simulink model "ofdm_txrx_mimo_2x"  (Simulink 6.6)
settings: Solver=VariableStepDiscrete  StartTime=0.0  StopTime=20e3  RelTol=1e-3
PreLoadFcn:
  | ofdm_rx_mimo_init
InitFcn:
  | ofdm_rx_mimo_init
StartFcn:
  | ofdm_rx_mimo_init
values from neighbouring files (symbols this model uses):
  BER_RAM_init_size = 2048    [ofdm_tx_mimo_init.m]
  CPLength = 16    [ofdm_tx_mimo_init.m]
  CRCPolynomial = hex2dec('04c11db7')    [ofdm_tx_mimo_init.m]
  freqOffset_track_filtCoef_i = hex2dec('2500')/2^32    [ofdm_rx_mimo_init.m]
  freqOffset_track_filtCoef_p = hex2dec('d0000')/2^32    [ofdm_rx_mimo_init.m]
  hi = zeros(1,length(cci))    [ofdm_rx_mimo_init.m]
  hr = zeros(1,length(ccr))    [ofdm_rx_mimo_init.m]
  max_CP_length = 16    [ofdm_tx_mimo_init.m]
  max_num_baseRateSymbols = 31    [ofdm_tx_mimo_init.m]
  max_num_subcarriers = 64    [ofdm_tx_mimo_init.m]
  max_num_trainingSymbols = 15    [ofdm_tx_mimo_init.m]
  max_numBytes = 16384    [ofdm_tx_mimo_init.m]
  max_OFDM_symbols = 2047    [ofdm_tx_mimo_init.m]
  modConstellation_qam16 = .75*[1 3 -1 -3]./3    [ofdm_tx_mimo_init.m]
  modConstellation_qam256 = 0.9375*[3 1 5 7 11 9 13 15 -3 -1 -5 -7 -11 -9 -13 -15]./15    [ofdm_tx_mimo_init.m]
  modConstellation_qam64 = 0.875*[3 1 5 7 -3 -1 -5 -7]./7    [ofdm_tx_mimo_init.m]
  modConstellation_qpsk = [1 -1]./sqrt(2)    [ofdm_tx_mimo_init.m]
  numBaseRateSymbols = 2    [ofdm_tx_mimo_init.m]
  numSubcarriers = 64    [ofdm_tx_mimo_init.m]
  numTrainingSymbols = 2    [ofdm_tx_mimo_init.m]
  pilot1_indicies = 7 + ( (64-7) * 2^16)    (= 3735559)    [ofdm_tx_mimo_init.m]
  pilot2_indicies = 21 + ( (64-21) * 2^16)    (= 2818069)    [ofdm_tx_mimo_init.m]
  pilotValue_neg = hex2dec('8000') + (2^16 * 0)    [ofdm_tx_mimo_init.m]
  pilotValue_pos = hex2dec('7FFF') + (2^16 * 0)    [ofdm_tx_mimo_init.m]
  pkt_crossCorr_thresh = 0.7    [ofdm_rx_mimo_init.m]
  pkt_energy_thresh = 0    [ofdm_rx_mimo_init.m]
  pkt_numPayloadBytes = 1400+42-6    (= 1436)    [ofdm_tx_mimo_init.m]
  pktByteNums = numHeaderBytes +  				byteIndex_numPayloadBytes(1)*2^8 +  				byteIndex_numPayloadBytes(2)*2^16 +  				byteIndex_simpleDynModMasks * 2^24    [ofdm_rx_mimo_init.m]
  PktDet_Delay = 26 + Symbol_Timing_Offset*2^7    (= 1050)    [ofdm_rx_mimo_init.m]
  preamble = 6*[0 shortsyms_10 longsyms_2]    [ofdm_tx_mimo_init.m]
  preamble_I = real(preamble)    [ofdm_tx_mimo_init.m]
  preamble_Q = imag(preamble)    [ofdm_tx_mimo_init.m]
  RAM_init_size = max_numBytes    (= 16384)    [ofdm_tx_mimo_init.m]
  reg_Interrupt_PktBuf_Control = reg_InterruptControl +  	1 * 2^16 +  	0 * 2^24    (= 65656)    [ofdm_rx_mimo_init.m]
  rx_controlBits = 1 * 2  		 + 1 * 4  		 + 0 * 8  		 + 1 * 16 * rx_SISO_Mode  		 + 1 * 32  		 + 1 * 64  		 + 0 * 128  		 + 1 * 256  		 + 0 * 512  		 + 1 * 1024  		 + 0 * 2048  		 + 1 * 4096  		 + 1 * 8192  		 + 0 * 16384  		 + 1 * 65536  		 + 1 * 131072  		 + 0 * 262144  		 + 1 * 524288    [ofdm_rx_mimo_init.m]
  Rx_PhaseNoiseTrack_K = 0.6094    [ofdm_rx_mimo_init.m]
  rx_postEq_scaling = round(rxScaling*2^11) * (1 + 2^16)    [ofdm_rx_mimo_init.m]
  RxDataScrambling_Seq = [40 198 78 63 82 173 102 245 48 111 172 115 147 230 216 93 72 65 62 2 205 242 122 90 128 83 105 97 73 10 5 252]    [ofdm_tx_mimo_init.m]
  simOnly_numSamples = length(preamble)+( (numSubcarriers+CPLength)*(numTrainingSymbols + numBaseRateSymbols + numFullRateSymbols/2) )    [ofdm_tx_mimo_init.m]
  train = [train train]    [ofdm_tx_mimo_init.m]
  tx_controlBits = (antB_preambleShift * 2^4) + (2^0 * tx_SISO_Mode) + 4*0 + 8*1    (= 73)    [ofdm_tx_mimo_init.m]
  TxDataScrambling_Seq = [40 198 78 63 82 173 102 245 48 111 172 115 147 230 216 93 72 65 62 2 205 242 122 90 128 83 105 97 73 10 5 252]    [ofdm_tx_mimo_init.m]
  txReg_symbolCounts = (2^16 * numFullRateSymbols) + (2^8 *numBaseRateSymbols ) + numTrainingSymbols    [ofdm_tx_mimo_init.m]
  TxRx_FFTScaling = bin2dec('010111') + (bin2dec('000101') * 2^6)    [ofdm_tx_mimo_init.m]
undefined symbols (not defined in the file or in its folder): AntA, AntB, BaseRate, CRC_table_gen, gcbh, intfilt, pktTiming_count_payloadStart, pktTiming_symbol_length, xlBlockLoadCallback, xlGetSimulinkPeriod
libraries: xbsIndex_r4 x3973, dspsigops x4, commchan3 x2, commrflib2 x2, dspsrcs4 x1
(part 22 of 38)

== system ofdm_txrx_mimo_2x/OFDM Rx MIMO/Equalizer & Packetizer/Equalizer/YB*[-HBA,HAA]/ComplexMult_x1 ==
  Re[A] : Inport
  Im[A] : Inport  Port=2
  Re[B] : Inport  Port=3
  Im[B] : Inport  Port=4
  Add1 : xbsIndex_r4/AddSub  mode=Addition  use_carryin=off  use_carryout=off  en=off  latency=0  precision=User Defined  arith_type="Signed  (2's comp)"  n_bits=18  bin_pt=16  quantization=Truncate  overflow=Flag as error  dbl_ovrd=off  use_behavioral_HDL=on  pipelined=off  use_rpm=on  hw_selection=Fabric  block_type=addsub
      settings: xl_area=[9 0 0 16 0 0 0]  block_version=VER_STRING_GOES_HERE
  Add2 : xbsIndex_r4/AddSub  mode=Addition  use_carryin=off  use_carryout=off  en=off  latency=0  precision=User Defined  arith_type="Signed  (2's comp)"  n_bits=18  bin_pt=16  quantization=Truncate  overflow=Flag as error  dbl_ovrd=off  use_behavioral_HDL=on  pipelined=off  use_rpm=on  hw_selection=Fabric  block_type=addsub
      settings: xl_area=[9 0 0 16 0 0 0]  block_version=VER_STRING_GOES_HERE
  Counter1 : xbsIndex_r4/Counter  Orientation=down  cnt_type=Free Running  cnt_to=4  operation=Up  start_count=0  cnt_by_val=1  arith_type=Unsigned  n_bits=1  bin_pt=0  load_pin=off  rst=off  en=off  explicit_period=on  period=1  dbl_ovrd=off  use_behavioral_HDL=off  use_rpm=on  implementation=Fabric  block_type=counter
      settings: xl_area=[3 3 0 4 0 0 0]  block_version=VER_STRING_GOES_HERE
  Delay3 : xbsIndex_r4/Delay  en=off  latency=1  dbl_ovrd=off  reg_retiming=off  block_type=delay
      settings: infoedit=Hardware notes: A delay line is a chain, each link of which is an SRL16 followed by a flip-flop. If register retiming is enabled, the delay line is a chain of flip-flops.  xl_area=[8 16 0 0 0 0 0]  block_version=VER_STRING_GOES_HERE
  Delay5 : xbsIndex_r4/Delay  en=off  latency=1  dbl_ovrd=off  reg_retiming=off  block_type=delay
      settings: infoedit=Hardware notes: A delay line is a chain, each link of which is an SRL16 followed by a flip-flop. If register retiming is enabled, the delay line is a chain of flip-flops.  xl_area=[8 16 0 0 0 0 0]  block_version=VER_STRING_GOES_HERE
  Down Sample1 : xbsIndex_r4/Down Sample  sample_ratio=2  sample_phase=First Value of Frame  en=off  latency=1  dbl_ovrd=off  block_type=dsamp
      settings: xl_area=[9 17 0 0 0 0 0]  block_version=VER_STRING_GOES_HERE
  Down Sample3 : xbsIndex_r4/Down Sample  sample_ratio=2  sample_phase=First Value of Frame  en=off  latency=1  dbl_ovrd=off  block_type=dsamp
      settings: xl_area=[9 17 0 0 0 0 0]  block_version=VER_STRING_GOES_HERE
  Mult3 : xbsIndex_r4/Mult  precision=User Defined  arith_type="Signed  (2's comp)"  n_bits=19  bin_pt=17  quantization=Truncate  overflow=Flag as error  en=off  latency=1  dbl_ovrd=off  use_behavioral_HDL=off  use_embedded=on  opt=Speed  optimum_pipeline=off  pipeline=off  use_rpm=on  placement_style=Rectangular shape  block_type=mult
      settings: infoedit=Hardware notes: To use the internal pipeline stage of the dedicated multiplier you must select 'Pipeline for maximum performance'.  xl_area=[14 27 0 27 0 1 0]  block_version=VER_STRING_GOES_HERE
  Mult4 : xbsIndex_r4/Mult  precision=User Defined  arith_type="Signed  (2's comp)"  n_bits=19  bin_pt=17  quantization=Truncate  overflow=Flag as error  en=off  latency=1  dbl_ovrd=off  use_behavioral_HDL=off  use_embedded=on  opt=Speed  optimum_pipeline=off  pipeline=off  use_rpm=on  placement_style=Rectangular shape  block_type=mult
      settings: infoedit=Hardware notes: To use the internal pipeline stage of the dedicated multiplier you must select 'Pipeline for maximum performance'.  xl_area=[14 27 0 27 0 1 0]  block_version=VER_STRING_GOES_HERE
  Mux{2..5} (4 alike) : xbsIndex_r4/Mux  inputs=2  en=off  latency=0  precision=Full  arith_type="Signed  (2's comp)"  n_bits=16  bin_pt=13  quantization=Truncate  overflow=Wrap  dbl_ovrd=off  block_type=mux
      settings: xl_area=[14 0 0 28 0 0 0]  block_version=VER_STRING_GOES_HERE
  Negate2 : xbsIndex_r4/Negate  precision=Full  arith_type="Signed  (2's comp)"  n_bits=14  bin_pt=13  quantization=Truncate  overflow=Flag as error  en=off  latency=0  dbl_ovrd=off  block_type=negate
      settings: xl_area=[7 0 0 14 0 0 0]  block_version=VER_STRING_GOES_HERE
  Up Sample{5..8} (4 alike) : xbsIndex_r4/Up Sample  sample_ratio=2  copy_samples=on  dbl_ovrd=off  block_type=usamp
      settings: infoedit="Up samples input data.  Inserted values  can be zeros or copies of the most recent input sample.<P><P>Hardware notes: No hardware is needed if inserted values are copies of the input sample; otherwise, a mux and single bit flip-flop are used."  xl_area=[0 0 0 0 0 0 0]  block_version=VER_STRING_GOES_HERE
  Re : Outport
  Im : Outport  Port=2
  wires:
  Counter1/1 -> Mux3/1, Mux2/1, Mux5/1, Mux4/1
  Delay3/1 -> Add2/2
  Mult3/1 -> Add2/1, Delay3/1
  Up Sample5/1 -> Negate2/1, Mux5/3
  Up Sample6/1 -> Mux2/3, Mux4/2
  Mux3/1 -> Mult3/1
  Mux2/1 -> Mult3/2
  Mux5/1 -> Mult4/1
  Mux4/1 -> Mult4/2
  Up Sample7/1 -> Mux3/2, Mux5/2
  Up Sample8/1 -> Mux2/2, Mux4/3
  Re[A]/1 -> Up Sample7/1
  Im[A]/1 -> Up Sample5/1
  Re[B]/1 -> Up Sample8/1
  Im[B]/1 -> Up Sample6/1
  Negate2/1 -> Mux3/3
  Mult4/1 -> Delay5/1, Add1/1
  Delay5/1 -> Add1/2
  Add1/1 -> Down Sample1/1
  Add2/1 -> Down Sample3/1
  Down Sample3/1 -> Re/1
  Down Sample1/1 -> Im/1

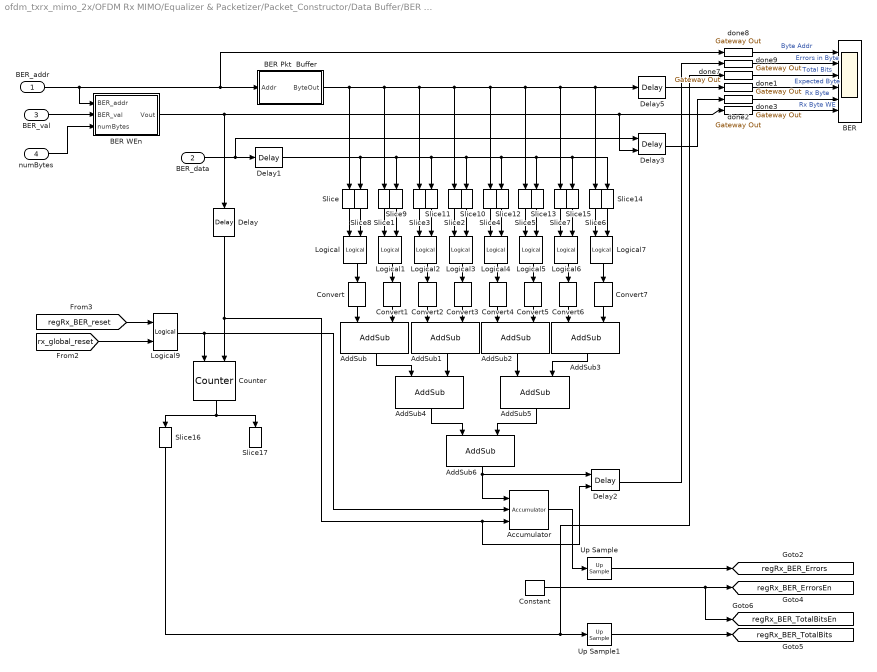
== system ofdm_txrx_mimo_2x/OFDM Rx MIMO/Equalizer & Packetizer/Packet_Constructor/Data Buffer/BER Calc ==
  BER_addr : Inport
  BER_data : Inport  Port=2
  BER_val : Inport  Port=3
  numBytes : Inport  Port=4
  Accumulator : xbsIndex_r4/Accumulator  operation=Add  n_bits=32  overflow=Wrap  scale=1  rst=on  hasbypass=off  en=on  dbl_ovrd=off  use_behavioral_HDL=on  implementation=Fabric  block_type=accum
      settings: infoedit="Adder or subtractor-based accumulator.   Output type and binary point position match the input.<P><P>Hardware notes: When \"Reinitialize with input 'b' on reset\" is selected, the accumulator is forced to run at the system rate even if the input 'b' is running at a slower rate."  xl_area=[0, 0, 0, 0, 0, 0, 0]  block_version=VER_STRING_GOES_HERE
  AddSub{,1..6} (7 alike) : xbsIndex_r4/AddSub  Orientation=down  mode=Addition  use_carryin=off  use_carryout=off  en=off  latency=0  precision=Full  arith_type=Unsigned  n_bits=8  bin_pt=2  quantization=Truncate  overflow=Wrap  dbl_ovrd=off  use_behavioral_HDL=on  pipelined=off  use_rpm=on  hw_selection=Fabric  block_type=addsub
      settings: xl_area=[0, 0, 0, 0, 0, 0, 0]  block_version=VER_STRING_GOES_HERE
  BER : Scope  NumInputPorts=6
      settings: TimeRange=7000  YMin=0~0~0~0~0~0  YMax=35~4~250~70~250~1  SaveName=ScopeData57
  BER Pkt  Buffer : Subsystem
  BER WEn : Subsystem
  Constant : xbsIndex_r4/Constant  arith_type=Boolean  const=1  n_bits=16  bin_pt=14  explicit_period=off  period=1  equ=P=C  opselect=C  inp2=PCIN>>17  opr=+  inp1=P  carry=CIN  dbl_ovrd=off  block_type=constant
      settings: block_version=VER_STRING_GOES_HERE
  Convert{,1..7} (8 alike) : xbsIndex_r4/Convert  Orientation=down  arith_type=Unsigned  n_bits=1  bin_pt=0  quantization=Truncate  overflow=Wrap  latency=0  dbl_ovrd=off  pipeline=off  block_type=convert
      settings: xl_area=[0, 0, 0, 0, 0, 0, 0]  block_version=VER_STRING_GOES_HERE
  Counter : xbsIndex_r4/Counter  Orientation=down  cnt_type=Free Running  cnt_to=Inf  operation=Up  start_count=0  cnt_by_val=8  arith_type=Unsigned  n_bits=48  bin_pt=0  load_pin=off  rst=on  en=on  explicit_period=off  period=1  dbl_ovrd=off  use_behavioral_HDL=off  use_rpm=on  implementation=Fabric  block_type=counter
      settings: xl_area=[0, 0, 0, 0, 0, 0, 0]  block_version=VER_STRING_GOES_HERE
  Delay : xbsIndex_r4/Delay  Orientation=down  en=off  latency=1  dbl_ovrd=off  reg_retiming=off  block_type=delay
      settings: infoedit=Hardware notes: A delay line is a chain, each link of which is an SRL16 followed by a flip-flop. If register retiming is enabled, the delay line is a chain of flip-flops.  xl_area=[0, 0, 0, 0, 0, 0, 0]  block_version=VER_STRING_GOES_HERE
  Delay1 : xbsIndex_r4/Delay  en=off  latency=1  dbl_ovrd=off  reg_retiming=off  block_type=delay
      settings: infoedit=Hardware notes: A delay line is a chain, each link of which is an SRL16 followed by a flip-flop. If register retiming is enabled, the delay line is a chain of flip-flops.  xl_area=[0, 0, 0, 0, 0, 0, 0]  block_version=VER_STRING_GOES_HERE
  Delay2 : xbsIndex_r4/Delay  en=on  latency=1  dbl_ovrd=off  reg_retiming=off  block_type=delay
      settings: infoedit=Hardware notes: A delay line is a chain, each link of which is an SRL16 followed by a flip-flop. If register retiming is enabled, the delay line is a chain of flip-flops.  xl_area=[0, 0, 0, 0, 0, 0, 0]  block_version=VER_STRING_GOES_HERE
  Delay3 : xbsIndex_r4/Delay  en=on  latency=1  dbl_ovrd=off  reg_retiming=off  block_type=delay
      settings: infoedit=Hardware notes: A delay line is a chain, each link of which is an SRL16 followed by a flip-flop. If register retiming is enabled, the delay line is a chain of flip-flops.  xl_area=[0, 0, 0, 0, 0, 0, 0]  block_version=VER_STRING_GOES_HERE
  Delay5 : xbsIndex_r4/Delay  en=off  latency=1  dbl_ovrd=off  reg_retiming=off  block_type=delay
      settings: infoedit=Hardware notes: A delay line is a chain, each link of which is an SRL16 followed by a flip-flop. If register retiming is enabled, the delay line is a chain of flip-flops.  xl_area=[0, 0, 0, 0, 0, 0, 0]  block_version=VER_STRING_GOES_HERE
  Logical{,1..7} (8 alike) : xbsIndex_r4/Logical  Orientation=down  logical_function=XOR  inputs=2  en=off  latency=0  precision=Full  arith_type=Unsigned  n_bits=1  bin_pt=0  align_bp=on  dbl_ovrd=off  block_type=logical
      settings: xl_area=[0, 0, 0, 0, 0, 0, 0]  block_version=VER_STRING_GOES_HERE
  Logical9 : xbsIndex_r4/Logical  logical_function=OR  inputs=2  en=off  latency=0  precision=Full  arith_type=Unsigned  n_bits=8  bin_pt=2  align_bp=on  dbl_ovrd=off  block_type=logical
      settings: xl_area=[4 0 0 4 0 0 0]  block_version=VER_STRING_GOES_HERE
  Slice : xbsIndex_r4/Slice  Orientation=down  nbits=1  boolean_output=on  mode=Upper Bit Location + Width  bit1=0  base1=MSB of Input  bit0=0  base0=LSB of Input  dbl_ovrd=off  block_type=slice
      settings: infoedit="Extracts a given range of bits from each input sample and presents it at the output.  The output type is ordinarily unsigned with binary point at zero, but can be Boolean when the slice is one bit wide.<P><P>Hardware notes: In hardware this block costs nothing."  block_version=VER_STRING_GOES_HERE
  Slice1 : xbsIndex_r4/Slice  Orientation=down  nbits=1  boolean_output=on  mode=Upper Bit Location + Width  bit1=-1  base1=MSB of Input  bit0=0  base0=LSB of Input  dbl_ovrd=off  block_type=slice
      settings: infoedit="Extracts a given range of bits from each input sample and presents it at the output.  The output type is ordinarily unsigned with binary point at zero, but can be Boolean when the slice is one bit wide.<P><P>Hardware notes: In hardware this block costs nothing."  block_version=VER_STRING_GOES_HERE
  Slice10 : xbsIndex_r4/Slice  Orientation=down  nbits=1  boolean_output=on  mode=Upper Bit Location + Width  bit1=-3  base1=MSB of Input  bit0=0  base0=LSB of Input  dbl_ovrd=off  block_type=slice
      settings: infoedit="Extracts a given range of bits from each input sample and presents it at the output.  The output type is ordinarily unsigned with binary point at zero, but can be Boolean when the slice is one bit wide.<P><P>Hardware notes: In hardware this block costs nothing."  block_version=VER_STRING_GOES_HERE
  Slice11 : xbsIndex_r4/Slice  Orientation=down  nbits=1  boolean_output=on  mode=Upper Bit Location + Width  bit1=-2  base1=MSB of Input  bit0=0  base0=LSB of Input  dbl_ovrd=off  block_type=slice
      settings: infoedit="Extracts a given range of bits from each input sample and presents it at the output.  The output type is ordinarily unsigned with binary point at zero, but can be Boolean when the slice is one bit wide.<P><P>Hardware notes: In hardware this block costs nothing."  block_version=VER_STRING_GOES_HERE
  Slice12 : xbsIndex_r4/Slice  Orientation=down  nbits=1  boolean_output=on  mode=Upper Bit Location + Width  bit1=-4  base1=MSB of Input  bit0=0  base0=LSB of Input  dbl_ovrd=off  block_type=slice
      settings: infoedit="Extracts a given range of bits from each input sample and presents it at the output.  The output type is ordinarily unsigned with binary point at zero, but can be Boolean when the slice is one bit wide.<P><P>Hardware notes: In hardware this block costs nothing."  block_version=VER_STRING_GOES_HERE
  Slice13 : xbsIndex_r4/Slice  Orientation=down  nbits=1  boolean_output=on  mode=Upper Bit Location + Width  bit1=-5  base1=MSB of Input  bit0=0  base0=LSB of Input  dbl_ovrd=off  block_type=slice
      settings: infoedit="Extracts a given range of bits from each input sample and presents it at the output.  The output type is ordinarily unsigned with binary point at zero, but can be Boolean when the slice is one bit wide.<P><P>Hardware notes: In hardware this block costs nothing."  block_version=VER_STRING_GOES_HERE
  Slice14 : xbsIndex_r4/Slice  Orientation=down  nbits=1  boolean_output=on  mode=Upper Bit Location + Width  bit1=-7  base1=MSB of Input  bit0=0  base0=LSB of Input  dbl_ovrd=off  block_type=slice
      settings: infoedit="Extracts a given range of bits from each input sample and presents it at the output.  The output type is ordinarily unsigned with binary point at zero, but can be Boolean when the slice is one bit wide.<P><P>Hardware notes: In hardware this block costs nothing."  block_version=VER_STRING_GOES_HERE
  Slice15 : xbsIndex_r4/Slice  Orientation=down  nbits=1  boolean_output=on  mode=Upper Bit Location + Width  bit1=-6  base1=MSB of Input  bit0=0  base0=LSB of Input  dbl_ovrd=off  block_type=slice
      settings: infoedit="Extracts a given range of bits from each input sample and presents it at the output.  The output type is ordinarily unsigned with binary point at zero, but can be Boolean when the slice is one bit wide.<P><P>Hardware notes: In hardware this block costs nothing."  block_version=VER_STRING_GOES_HERE
  Slice16 : xbsIndex_r4/Slice  Orientation=down  nbits=32  boolean_output=off  mode=Lower Bit Location + Width  bit1=0  base1=MSB of Input  bit0=0  base0=LSB of Input  dbl_ovrd=off  block_type=slice
      settings: infoedit="Extracts a given range of bits from each input sample and presents it at the output.  The output type is ordinarily unsigned with binary point at zero, but can be Boolean when the slice is one bit wide.<P><P>Hardware notes: In hardware this block costs nothing."  block_version=VER_STRING_GOES_HERE
  Slice17 : xbsIndex_r4/Slice  Orientation=down  nbits=16  boolean_output=off  mode=Upper Bit Location + Width  bit1=0  base1=MSB of Input  bit0=0  base0=LSB of Input  dbl_ovrd=off  block_type=slice
      settings: infoedit="Extracts a given range of bits from each input sample and presents it at the output.  The output type is ordinarily unsigned with binary point at zero, but can be Boolean when the slice is one bit wide.<P><P>Hardware notes: In hardware this block costs nothing."  block_version=VER_STRING_GOES_HERE
  Slice2 : xbsIndex_r4/Slice  Orientation=down  nbits=1  boolean_output=on  mode=Upper Bit Location + Width  bit1=-3  base1=MSB of Input  bit0=0  base0=LSB of Input  dbl_ovrd=off  block_type=slice
      settings: infoedit="Extracts a given range of bits from each input sample and presents it at the output.  The output type is ordinarily unsigned with binary point at zero, but can be Boolean when the slice is one bit wide.<P><P>Hardware notes: In hardware this block costs nothing."  block_version=VER_STRING_GOES_HERE
  Slice3 : xbsIndex_r4/Slice  Orientation=down  nbits=1  boolean_output=on  mode=Upper Bit Location + Width  bit1=-2  base1=MSB of Input  bit0=0  base0=LSB of Input  dbl_ovrd=off  block_type=slice
      settings: infoedit="Extracts a given range of bits from each input sample and presents it at the output.  The output type is ordinarily unsigned with binary point at zero, but can be Boolean when the slice is one bit wide.<P><P>Hardware notes: In hardware this block costs nothing."  block_version=VER_STRING_GOES_HERE
  Slice4 : xbsIndex_r4/Slice  Orientation=down  nbits=1  boolean_output=on  mode=Upper Bit Location + Width  bit1=-4  base1=MSB of Input  bit0=0  base0=LSB of Input  dbl_ovrd=off  block_type=slice
      settings: infoedit="Extracts a given range of bits from each input sample and presents it at the output.  The output type is ordinarily unsigned with binary point at zero, but can be Boolean when the slice is one bit wide.<P><P>Hardware notes: In hardware this block costs nothing."  block_version=VER_STRING_GOES_HERE
  Slice5 : xbsIndex_r4/Slice  Orientation=down  nbits=1  boolean_output=on  mode=Upper Bit Location + Width  bit1=-5  base1=MSB of Input  bit0=0  base0=LSB of Input  dbl_ovrd=off  block_type=slice
      settings: infoedit="Extracts a given range of bits from each input sample and presents it at the output.  The output type is ordinarily unsigned with binary point at zero, but can be Boolean when the slice is one bit wide.<P><P>Hardware notes: In hardware this block costs nothing."  block_version=VER_STRING_GOES_HERE
  Slice6 : xbsIndex_r4/Slice  Orientation=down  nbits=1  boolean_output=on  mode=Upper Bit Location + Width  bit1=-7  base1=MSB of Input  bit0=0  base0=LSB of Input  dbl_ovrd=off  block_type=slice
      settings: infoedit="Extracts a given range of bits from each input sample and presents it at the output.  The output type is ordinarily unsigned with binary point at zero, but can be Boolean when the slice is one bit wide.<P><P>Hardware notes: In hardware this block costs nothing."  block_version=VER_STRING_GOES_HERE
  Slice7 : xbsIndex_r4/Slice  Orientation=down  nbits=1  boolean_output=on  mode=Upper Bit Location + Width  bit1=-6  base1=MSB of Input  bit0=0  base0=LSB of Input  dbl_ovrd=off  block_type=slice
      settings: infoedit="Extracts a given range of bits from each input sample and presents it at the output.  The output type is ordinarily unsigned with binary point at zero, but can be Boolean when the slice is one bit wide.<P><P>Hardware notes: In hardware this block costs nothing."  block_version=VER_STRING_GOES_HERE
  Slice8 : xbsIndex_r4/Slice  Orientation=down  nbits=1  boolean_output=on  mode=Upper Bit Location + Width  bit1=0  base1=MSB of Input  bit0=0  base0=LSB of Input  dbl_ovrd=off  block_type=slice
      settings: infoedit="Extracts a given range of bits from each input sample and presents it at the output.  The output type is ordinarily unsigned with binary point at zero, but can be Boolean when the slice is one bit wide.<P><P>Hardware notes: In hardware this block costs nothing."  block_version=VER_STRING_GOES_HERE
  Slice9 : xbsIndex_r4/Slice  Orientation=down  nbits=1  boolean_output=on  mode=Upper Bit Location + Width  bit1=-1  base1=MSB of Input  bit0=0  base0=LSB of Input  dbl_ovrd=off  block_type=slice
      settings: infoedit="Extracts a given range of bits from each input sample and presents it at the output.  The output type is ordinarily unsigned with binary point at zero, but can be Boolean when the slice is one bit wide.<P><P>Hardware notes: In hardware this block costs nothing."  block_version=VER_STRING_GOES_HERE
  Up Sample : xbsIndex_r4/Up Sample  sample_ratio=2  copy_samples=on  dbl_ovrd=off  block_type=usamp
      settings: infoedit="Up samples input data.  Inserted values  can be zeros or copies of the most recent input sample.<P><P>Hardware notes: No hardware is needed if inserted values are copies of the input sample; otherwise, a mux and single bit flip-flop are used."  block_version=VER_STRING_GOES_HERE
  Up Sample1 : xbsIndex_r4/Up Sample  sample_ratio=2  copy_samples=on  dbl_ovrd=off  block_type=usamp
      settings: infoedit="Up samples input data.  Inserted values  can be zeros or copies of the most recent input sample.<P><P>Hardware notes: No hardware is needed if inserted values are copies of the input sample; otherwise, a mux and single bit flip-flop are used."  block_version=VER_STRING_GOES_HERE
  done{1..3,7..9} (6 alike) : xbsIndex_r4/Gateway Out  hdl_port=off  timing_constraint=None  locs_specified=off  LOCs={}  block_type=gatewayout
      settings: infoedit="Gateway out block.  Converts Xilinx fixed point inputs into ouputs of type Simulink integer, double, or fixed point.<P><P>Hardware notes:  In hardware these blocks become top level output ports or are discarded, depending on how they are configured."  xl_area=[0 0 0 0 1 0 0]  block_version=VER_STRING_GOES_HERE
  tag scope: regRx_BER_Errors=global, regRx_BER_ErrorsEn=global, regRx_BER_TotalBits=global, regRx_BER_TotalBitsEn=global
  wires:
  Delay/1 -> Counter/2, Accumulator/3, Delay2/2
  Slice16/1 -> Up Sample1/1, done7/1
  Counter/1 -> Slice17/1, Slice16/1
  BER WEn/1(Vout) -> done3/1, Delay3/2, Delay/1
  Convert7/1 -> AddSub3/2
  Convert6/1 -> AddSub3/1
  Convert5/1 -> AddSub2/2
  Convert4/1 -> AddSub2/1
  Convert3/1 -> AddSub1/2
  Convert2/1 -> AddSub1/1
  Convert1/1 -> AddSub/2
  Convert/1 -> AddSub/1
  Accumulator/1 -> Up Sample/1
  AddSub6/1 -> Accumulator/1, Delay2/1
  AddSub5/1 -> AddSub6/2
  AddSub4/1 -> AddSub6/1
  AddSub1/1 -> AddSub4/2
  AddSub/1 -> AddSub4/1
  AddSub3/1 -> AddSub5/2
  AddSub2/1 -> AddSub5/1
  Logical7/1 -> Convert7/1
  Logical6/1 -> Convert6/1
  Logical5/1 -> Convert5/1
  Logical4/1 -> Convert4/1
  Logical3/1 -> Convert3/1
  Logical2/1 -> Convert2/1
  Logical1/1 -> Convert1/1
  Logical/1 -> Convert/1
  Slice14/1 -> Logical7/2
  Slice6/1 -> Logical7/1
  Slice15/1 -> Logical6/2
  Slice7/1 -> Logical6/1
  Slice13/1 -> Logical5/2
  Slice5/1 -> Logical5/1
  Slice12/1 -> Logical4/2
  Slice4/1 -> Logical4/1
  Slice10/1 -> Logical3/2
  Slice2/1 -> Logical3/1
  Slice11/1 -> Logical2/2
  Slice3/1 -> Logical2/1
  Slice9/1 -> Logical1/2
  Slice1/1 -> Logical1/1
  Slice8/1 -> Logical/2
  Slice/1 -> Logical/1
  BER_data/1 -> Delay1/1, Delay3/1
  BER_addr/1 -> done8/1, BER Pkt  Buffer/1(Addr), BER WEn/1(BER_addr)
  [regRx_BER_reset] -> Logical9/1
  Logical9/1 -> Counter/1, Accumulator/2
  [rx_global_reset] -> Logical9/2
  Constant/1 -> [regRx_BER_ErrorsEn], [regRx_BER_TotalBitsEn]
  Up Sample/1 -> [regRx_BER_Errors]
  Up Sample1/1 -> [regRx_BER_TotalBits]
  Delay1/1 -> Slice11/1, Slice15/1, Slice14/1, Slice13/1, Slice12/1, Slice10/1, Slice9/1, Slice8/1
  Delay2/1 -> done9/1
  BER Pkt  Buffer/1(ByteOut) -> Slice1/1, Slice3/1, Slice2/1, Slice4/1, Slice5/1, Slice7/1, Slice6/1, Delay5/1, Slice/1
  done8/1 -> BER/1  "Byte Addr"
  done9/1 -> BER/2  "Errors in Byte"
  done7/1 -> BER/3  "Total Bits"
  done1/1 -> BER/4  "Expected Byte"
  done2/1 -> BER/5  "Rx Byte"
  done3/1 -> BER/6  "Rx Byte WE"
  Delay3/1 -> done2/1
  Delay5/1 -> done1/1
  BER_val/1 -> BER WEn/2(BER_val)
  numBytes/1 -> BER WEn/3(numBytes)

== system ofdm_txrx_mimo_2x/OFDM Rx MIMO/Equalizer & Packetizer/Packet_Constructor/Data Buffer/Descrambling & Pkt Buffer ==
  Addr : Inport
  Data : Inport  Port=2
  We : Inport  Port=3
  BRAM WEn Decoding : Subsystem
  DataReplication : Subsystem
  De-Scrambling : Subsystem
  Error Injector (Sim Only!) : Subsystem
  Gateway Out{1,3..5} (4 alike) : xbsIndex_r4/Gateway Out  hdl_port=off  timing_constraint=None  locs_specified=off  LOCs={}  block_type=gatewayout
      settings: infoedit="Gateway out block.  Converts Xilinx fixed point inputs into ouputs of type Simulink integer, double, or fixed point.<P><P>Hardware notes:  In hardware these blocks become top level output ports or are discarded, depending on how they are configured."  xl_area=[0, 0, 0, 0, 0, 0, 0]  block_version=VER_STRING_GOES_HERE
  MSB : xbsIndex_r4/Slice  nbits=log2(max_numBytes)-3  boolean_output=off  mode=Upper Bit Location + Width  bit1=0  base1=MSB of Input  bit0=0  base0=LSB of Input  dbl_ovrd=off  block_type=slice
      settings: infoedit="Extracts a given range of bits from each input sample and presents it at the output.  The output type is ordinarily unsigned with binary point at zero, but can be Boolean when the slice is one bit wide.<P><P>Hardware notes: In hardware this block costs nothing."  block_version=8.2.02
  Rx Data Descrambling : Scope  NumInputPorts=6
      settings: YMin=181~0~0~0~0~-1  YMax=192~275~1~275~250~1  SaveName=ScopeData27
  Up Sample{3..7} (5 alike) : xbsIndex_r4/Up Sample  sample_ratio=2  copy_samples=on  dbl_ovrd=off  block_type=usamp
      settings: infoedit="Up samples input data.  Inserted values  can be zeros or copies of the most recent input sample.<P><P>Hardware notes: No hardware is needed if inserted values are copies of the input sample; otherwise, a mux and single bit flip-flop are used."  block_version=8.2.02
  " Addr" : Outport
  " Data" : Outport  Port=2
  " Vout" : Outport  Port=3
  tag scope: BRAM_Rx_AnyWrEn=global, BRAM_Rx_DOut=global, BRAM_Rx_WrAddr=global, BRAM_Rx_WrEn=global, DebugBRAM_Rx_ByteOut=global
  wires:
  De-Scrambling/1(" Addr") -> Up Sample5/1, Up Sample7/1, " Addr"/1, Error Injector (Sim Only!)/1(ByteAddr)
  De-Scrambling/2(" Data") -> Gateway Out5/1, Up Sample3/1, " Data"/1, Error Injector (Sim Only!)/2(Data_I)
  De-Scrambling/3(" We") -> " Vout"/1, Up Sample4/1, Up Sample6/1
  We/1 -> Gateway Out4/1, De-Scrambling/3(We)
  Data/1 -> Gateway Out3/1, De-Scrambling/2(Data)
  Addr/1 -> Gateway Out1/1, De-Scrambling/1(Addr)
  Gateway Out1/1 -> Rx Data Descrambling/1
  Gateway Out3/1 -> Rx Data Descrambling/2
  Gateway Out4/1 -> Rx Data Descrambling/3
  Gateway Out5/1 -> Rx Data Descrambling/4
  DataReplication/1(8BytesOut) -> [BRAM_Rx_DOut]
  BRAM WEn Decoding/1(ByteEn) -> [BRAM_Rx_WrEn]
  MSB/1 -> [BRAM_Rx_WrAddr]
  Up Sample3/1 -> DataReplication/1(ByteIn), [DebugBRAM_Rx_ByteOut]
  Up Sample4/1 -> BRAM WEn Decoding/2(WrEn)
  Up Sample5/1 -> BRAM WEn Decoding/1(Byte Addr)
  Up Sample6/1 -> [BRAM_Rx_AnyWrEn]
  Up Sample7/1 -> MSB/1

== system ofdm_txrx_mimo_2x/OFDM Rx MIMO/Equalizer & Packetizer/Packet_Constructor/Data Buffer/Error Checking ==
  ByteIn : Inport
  Vin : Inport  Port=2
  ByteAddr : Inport  Port=3
  NumBytes : Inport  Port=4
  Rst : Inport  Port=5
  CRC Calc : Subsystem
  Error Checking : Subsystem
  Gateway Out{1..11} (11 alike) : xbsIndex_r4/Gateway Out  hdl_port=off  timing_constraint=None  locs_specified=off  LOCs={}  block_type=gatewayout
      settings: infoedit="Gateway out block.  Converts Xilinx fixed point inputs into ouputs of type Simulink integer, double, or fixed point.<P><P>Hardware notes:  In hardware these blocks become top level output ports or are discarded, depending on how they are configured."  xl_area=[0, 0, 0, 0, 0, 0, 0]  block_version=VER_STRING_GOES_HERE
  Posedge : Subsystem
  Relational : xbsIndex_r4/Relational  mode=a>=b  en=off  latency=0  dbl_ovrd=off  block_type=relational
      settings: xl_area=[0, 0, 0, 0, 0, 0, 0]  block_version=VER_STRING_GOES_HERE
  Rx CRC : Scope  NumInputPorts=6
      settings: YMin=0~0~-1~0~0~-1  YMax=175~1~1~4.5e+009~1~1  SaveName=ScopeData8
  Rx CRC Bytes : Scope  NumInputPorts=6
      settings: TimeRange=6000  YMin=0~0~0~0~-1~0  YMax=300~300~300~300~1~1  SaveName=ScopeData71
  PktError : Outport
  ResetRx : Outport  Port=2
  GoodHeader : Outport  Port=3
  BadHeader : Outport  Port=4
  wires:
  Vin/1 -> CRC Calc/2(Vin), Gateway Out11/1, Gateway Out1/1
  ByteIn/1 -> CRC Calc/1(ByteIn), Gateway Out6/1
  Rst/1 -> Error Checking/2(Rst), CRC Calc/3(Rst), Gateway Out3/1
  CRC Calc/1(32-bit CRC) -> Error Checking/1(32-bit CRC)
  Gateway Out1/1 -> Rx CRC/1
  Gateway Out3/1 -> Rx CRC/2
  Gateway Out4/1 -> Rx CRC/3
  Gateway Out5/1 -> Rx CRC/4
  Gateway Out2/1 -> Rx CRC/5
  Gateway Out11/1 -> Rx CRC Bytes/6
  ByteAddr/1 -> Relational/1, Gateway Out4/1, Error Checking/5(ByteAddr), Gateway Out8/1
  NumBytes/1 -> Relational/2, Gateway Out5/1, Error Checking/4(NumBytes), Gateway Out7/1
  Relational/1 -> Posedge/1(D)
  Posedge/1(Q) -> Error Checking/3(LastByte)
  Error Checking/1(PktError) -> PktError/1, Gateway Out2/1, Gateway Out9/1
  Gateway Out6/1 -> Rx CRC Bytes/1
  Gateway Out7/1 -> Rx CRC Bytes/2
  Gateway Out8/1 -> Rx CRC Bytes/3
  Gateway Out9/1 -> Rx CRC Bytes/4
  Gateway Out10/1 -> Rx CRC Bytes/5
  Error Checking/2(ResetRx) -> Gateway Out10/1, ResetRx/1
  Error Checking/3(GoodHeader) -> GoodHeader/1
  Error Checking/4(BadHeader) -> BadHeader/1

== system ofdm_txrx_mimo_2x/OFDM Rx MIMO/Equalizer & Packetizer/Packet_Constructor/Data Buffer/Header Decoding ==
  RxByteAddr : Inport
  RxByte : Inport  Port=2
  RxByteWE : Inport  Port=3
  Rst : Inport  Port=4
  4LSB : xbsIndex_r4/Slice  nbits=4  boolean_output=off  mode=Lower Bit Location + Width  bit1=0  base1=MSB of Input  bit0=0  base0=LSB of Input  dbl_ovrd=off  block_type=slice
      settings: infoedit="Extracts a given range of bits from each input sample and presents it at the output.  The output type is ordinarily unsigned with binary point at zero, but can be Boolean when the slice is one bit wide.<P><P>Hardware notes: In hardware this block costs nothing."  block_version=VER_STRING_GOES_HERE
  4MSB : xbsIndex_r4/Slice  nbits=4  boolean_output=off  mode=Upper Bit Location + Width  bit1=0  base1=MSB of Input  bit0=0  base0=LSB of Input  dbl_ovrd=off  block_type=slice
      settings: infoedit="Extracts a given range of bits from each input sample and presents it at the output.  The output type is ordinarily unsigned with binary point at zero, but can be Boolean when the slice is one bit wide.<P><P>Hardware notes: In hardware this block costs nothing."  block_version=VER_STRING_GOES_HERE
  8LSB : xbsIndex_r4/Slice  nbits=8  boolean_output=off  mode=Lower Bit Location + Width  bit1=0  base1=MSB of Input  bit0=0  base0=LSB of Input  dbl_ovrd=off  block_type=slice
      settings: infoedit="Extracts a given range of bits from each input sample and presents it at the output.  The output type is ordinarily unsigned with binary point at zero, but can be Boolean when the slice is one bit wide.<P><P>Hardware notes: In hardware this block costs nothing."  block_version=VER_STRING_GOES_HERE
  8LSB+8 : xbsIndex_r4/Slice  nbits=8  boolean_output=off  mode=Lower Bit Location + Width  bit1=0  base1=MSB of Input  bit0=8  base0=LSB of Input  dbl_ovrd=off  block_type=slice
      settings: infoedit="Extracts a given range of bits from each input sample and presents it at the output.  The output type is ordinarily unsigned with binary point at zero, but can be Boolean when the slice is one bit wide.<P><P>Hardware notes: In hardware this block costs nothing."  block_version=VER_STRING_GOES_HERE
  8MSB : xbsIndex_r4/Slice  nbits=8  boolean_output=off  mode=Upper Bit Location + Width  bit1=0  base1=MSB of Input  bit0=0  base0=LSB of Input  dbl_ovrd=off  block_type=slice
      settings: infoedit="Extracts a given range of bits from each input sample and presents it at the output.  The output type is ordinarily unsigned with binary point at zero, but can be Boolean when the slice is one bit wide.<P><P>Hardware notes: In hardware this block costs nothing."  block_version=VER_STRING_GOES_HERE
  Assert1 : xbsIndex_r4/Assert  assert_type=off  type_source=Explicitly  arith_type="Signed  (2's comp)"  n_bits=16  bin_pt=14  assert_rate=on  rate_source=Explicitly  period=2  output_port=on  block_type=assert
      settings: infoedit=Asserts a user-defined sample rate and/or type on a signal.<P><P>Hardware notes: In hardware this block costs nothing.  block_version=VER_STRING_GOES_HERE
  Assert2 : xbsIndex_r4/Assert  assert_type=off  type_source=Explicitly  arith_type="Signed  (2's comp)"  n_bits=16  bin_pt=14  assert_rate=on  rate_source=Explicitly  period=2  output_port=on  block_type=assert
      settings: infoedit=Asserts a user-defined sample rate and/or type on a signal.<P><P>Hardware notes: In hardware this block costs nothing.  block_version=VER_STRING_GOES_HERE
  Convert : xbsIndex_r4/Convert  arith_type=Boolean  n_bits=8  bin_pt=6  quantization=Truncate  overflow=Wrap  latency=0  dbl_ovrd=off  pipeline=off  block_type=convert
      settings: xl_area=[0, 0, 0, 0, 0, 0, 0]  block_version=VER_STRING_GOES_HERE
  Logical1 : xbsIndex_r4/Logical  logical_function=AND  inputs=2  en=off  latency=0  precision=Full  arith_type=Unsigned  n_bits=8  bin_pt=2  align_bp=on  dbl_ovrd=off  block_type=logical
      settings: xl_area=[1 0 0 1 0 0 0]  block_version=VER_STRING_GOES_HERE
  Logical4 : xbsIndex_r4/Logical  logical_function=AND  inputs=3  en=off  latency=0  precision=Full  arith_type=Unsigned  n_bits=8  bin_pt=2  align_bp=on  dbl_ovrd=off  block_type=logical
      settings: xl_area=[1 0 0 1 0 0 0]  block_version=VER_STRING_GOES_HERE
  Logical5 : xbsIndex_r4/Logical  logical_function=AND  inputs=3  en=off  latency=0  precision=Full  arith_type=Unsigned  n_bits=8  bin_pt=2  align_bp=on  dbl_ovrd=off  block_type=logical
      settings: xl_area=[1 0 0 1 0 0 0]  block_version=VER_STRING_GOES_HERE
  Register1 : xbsIndex_r4/Register  init=0%floor((pkt_numPayloadBytes/256))  rst=on  en=on  dbl_ovrd=off  block_type=register
      settings: xl_area=[5 10 0 0 0 0 0]  block_version=VER_STRING_GOES_HERE
  Relational1 : xbsIndex_r4/Relational  mode=a=b  en=off  latency=0  dbl_ovrd=off  block_type=relational
      settings: xl_area=[6 0 0 11 0 0 0]  block_version=VER_STRING_GOES_HERE
  Relational2 : xbsIndex_r4/Relational  mode=a=b  en=off  latency=0  dbl_ovrd=off  block_type=relational
      settings: xl_area=[6 0 0 11 0 0 0]  block_version=VER_STRING_GOES_HERE
  Relational3 : xbsIndex_r4/Relational  mode=a=b  en=off  latency=0  dbl_ovrd=off  block_type=relational
      settings: xl_area=[6 0 0 11 0 0 0]  block_version=VER_STRING_GOES_HERE
  Rx Bytes : Scope  NumInputPorts=5
      settings: TimeRange=1800  YMin=116~80~137.75~0~1.9  YMax=120~220~152.25~1~2.1  SaveName=ScopeData65
  done{1..10} (10 alike) : xbsIndex_r4/Gateway Out  hdl_port=off  timing_constraint=None  locs_specified=off  LOCs={}  block_type=gatewayout
      settings: infoedit="Gateway out block.  Converts Xilinx fixed point inputs into ouputs of type Simulink integer, double, or fixed point.<P><P>Hardware notes:  In hardware these blocks become top level output ports or are discarded, depending on how they are configured."  xl_area=[0 0 0 0 1 0 0]  block_version=VER_STRING_GOES_HERE
  modMasks : Display
  "numBytes " : Display
  numBytesUpdate : Subsystem
  numBytes_LSB : Display
  numBytes_MSB : Display
  numBytes_modMasks : Display
  numBytes : Outport
  modMask : Outport  Port=2
  wires:
  Convert/1 -> Logical5/3, Logical4/3
  [regRx_dynPktEn] -> Convert/1
  Relational3/1 -> Logical5/2
  8LSB+8/1 -> Relational3/2, done4/1
  Relational2/1 -> Logical4/2
  8LSB/1 -> Relational2/2, done3/1
  Rst/1 -> numBytesUpdate/1(rst), Register1/2
  Logical4/1 -> numBytesUpdate/3(lsb_EN)
  Logical5/1 -> numBytesUpdate/4(msb_EN)
  numBytesUpdate/1(numBytes) -> Assert1/1
  Assert1/1 -> numBytes/1, done1/1, done8/1
  [regRx_headerByteNums] -> 8LSB+8/1, 8LSB/1, 8MSB/1
  RxByteWE/1 -> Logical4/1, Logical5/1, Logical1/1, done2/1
  RxByte/1 -> numBytesUpdate/2(d), Register1/1, done7/1
  RxByteAddr/1 -> Relational2/1, Relational3/1, Relational1/1, done6/1
  Relational1/1 -> Logical1/2
  Logical1/1 -> Register1/3
  8MSB/1 -> Relational1/2, done5/1
  Register1/1 -> Assert2/1
  Assert2/1 -> modMask/1, 4MSB/1, 4LSB/1, done9/1, done10/1
  done6/1 -> Rx Bytes/1
  done7/1 -> Rx Bytes/2
  done1/1 -> Rx Bytes/3
  done9/1 -> Rx Bytes/5
  done2/1 -> Rx Bytes/4
  done3/1 -> numBytes_LSB/1
  done4/1 -> numBytes_MSB/1
  done5/1 -> numBytes_modMasks/1
  done8/1 -> "numBytes "/1
  done10/1 -> modMasks/1

== system ofdm_txrx_mimo_2x/OFDM Rx MIMO/Equalizer & Packetizer/Packet_Constructor/Modulation RAM/Addr Gen ==
  note: The modulation RAM stores 3x(numSubcarriers) values:
      | [0:numSc-1]: Masks for antenna A full rate symbols
      | [numSc:2*numSc-1]: Masks for antenna B full rate symbols
      | [2*numSc:3*numSc-1]: Masks for base rate symbols (same for both antennas)
  note: This block produces the correct memory address to lookup the modulation mask for a given subcarrier in a given OFDM symbol.
      | The LSB of the symbol index is treated as the antenna selector. During base rate symbols, this bit is forced to zero so that both
      | antennas use the same set of modulation masks for their base rate symbols.
  Xk_index : Inport
  Sym Index : Inport  Port=2
  1LSB : xbsIndex_r4/Slice  nbits=1  boolean_output=off  mode=Lower Bit Location + Width  bit1=0  base1=MSB of Input  bit0=0  base0=LSB of Input  dbl_ovrd=off  block_type=slice
      settings: infoedit="Extracts a given range of bits from each input sample and presents it at the output.  The output type is ordinarily unsigned with binary point at zero, but can be Boolean when the slice is one bit wide.<P><P>Hardware notes: In hardware this block costs nothing."  block_version=VER_STRING_GOES_HERE
  1b Zero : xbsIndex_r4/Constant  arith_type=Unsigned  const=0  n_bits=1  bin_pt=0  explicit_period=off  period=1  equ=P=C  opselect=C  inp2=PCIN>>17  opr=+  inp1=P  carry=CIN  dbl_ovrd=off  block_type=constant
      settings: block_version=VER_STRING_GOES_HERE
  Concat : xbsIndex_r4/Concat  num_inputs=2  dbl_ovrd=off  block_type=concat
      settings: block_version=VER_STRING_GOES_HERE
  Concat1 : xbsIndex_r4/Concat  num_inputs=3  dbl_ovrd=off  block_type=concat
      settings: block_version=VER_STRING_GOES_HERE
  Convert : xbsIndex_r4/Convert  arith_type=Unsigned  n_bits=1  bin_pt=0  quantization=Truncate  overflow=Wrap  latency=0  dbl_ovrd=off  pipeline=off  block_type=convert
      settings: block_version=VER_STRING_GOES_HERE
  Convert1 : xbsIndex_r4/Convert  arith_type=Unsigned  n_bits=1  bin_pt=0  quantization=Truncate  overflow=Wrap  latency=0  dbl_ovrd=off  pipeline=off  block_type=convert
      settings: block_version=VER_STRING_GOES_HERE
  Convert2 : xbsIndex_r4/Convert  arith_type=Boolean  n_bits=1  bin_pt=0  quantization=Truncate  overflow=Wrap  latency=0  dbl_ovrd=off  pipeline=off  block_type=convert
      settings: block_version=VER_STRING_GOES_HERE
  Inverter2 : xbsIndex_r4/Inverter  en=off  latency=0  dbl_ovrd=off  block_type=inv
      settings: block_version=VER_STRING_GOES_HERE
  Logical1 : xbsIndex_r4/Logical  logical_function=AND  inputs=2  en=off  latency=0  precision=Full  arith_type=Unsigned  n_bits=1  bin_pt=0  align_bp=on  dbl_ovrd=off  block_type=logical
      settings: block_version=VER_STRING_GOES_HERE
  Logical2 : xbsIndex_r4/Logical  logical_function=AND  inputs=2  en=off  latency=0  precision=Full  arith_type=Unsigned  n_bits=1  bin_pt=0  align_bp=on  dbl_ovrd=off  block_type=logical
      settings: block_version=VER_STRING_GOES_HERE
  Relational8 : xbsIndex_r4/Relational  mode=a>b  en=off  latency=0  dbl_ovrd=off  block_type=relational
      settings: xl_area=[2 0 0 4 0 0 0]  block_version=VER_STRING_GOES_HERE
  Rx Mod Sel Control : Scope  NumInputPorts=5
      settings: TimeRange=2000  YMin=16.15~-1~-1~-1~-1  YMax=17.85~1~1~1~1  SaveName=ScopeData36
  Shift : xbsIndex_r4/Shift  shift_dir=Left  shift_bits=1  en=off  latency=0  precision=Full  arith_type="Signed  (2's comp)"  n_bits=16  bin_pt=14  quantization=Truncate  overflow=Wrap  dbl_ovrd=off  block_type=shift
      settings: block_version=VER_STRING_GOES_HERE
  done{1..3,6..7} (5 alike) : xbsIndex_r4/Gateway Out  hdl_port=off  timing_constraint=None  locs_specified=off  LOCs={}  block_type=gatewayout
      settings: infoedit="Gateway out block.  Converts Xilinx fixed point inputs into ouputs of type Simulink integer, double, or fixed point.<P><P>Hardware notes:  In hardware these blocks become top level output ports or are discarded, depending on how they are configured."  xl_area=[0 0 0 0 1 0 0]  block_version=VER_STRING_GOES_HERE
  IgnoreSym : Outport
  Mod Addr : Outport  Port=2
  FullRate : Outport  Port=3
  wires:
  Shift/1 -> Relational8/1
  Sym Index/1 -> Relational8/2, 1LSB/1, done6/1
  Relational8/1 -> Inverter2/1, Convert/1, Logical2/1, done3/1
  Concat1/1 -> Mod Addr/1, done1/1
  Logical1/1 -> Convert1/1
  Xk_index/1 -> Concat1/3, done7/1
  1LSB/1 -> Convert2/1
  Inverter2/1 -> Logical1/1, FullRate/1
  Convert/1 -> Concat1/1
  Convert1/1 -> Concat1/2
  Convert2/1 -> Logical1/2, Logical2/2
  Concat/1 -> Shift/1
  1b Zero/1 -> Concat/1
  [regRx_numTrainPlusBaseRateSyms] -> Concat/2
  Logical2/1 -> IgnoreSym/1, done2/1
  done6/1 -> Rx Mod Sel Control/1
  done7/1 -> Rx Mod Sel Control/2
  done1/1 -> Rx Mod Sel Control/3
  done2/1 -> Rx Mod Sel Control/4
  done3/1 -> Rx Mod Sel Control/5

== system ofdm_txrx_mimo_2x/OFDM Rx MIMO/Equalizer & Packetizer/Packet_Constructor/Modulation RAM/Non-shared (read-only) Modulation Masks ==
  Addr : Inport
  Constant : xbsIndex_r4/Constant  arith_type=Unsigned  const=0  n_bits=4  bin_pt=0  explicit_period=off  period=1  equ=P=C  opselect=C  inp2=PCIN>>17  opr=+  inp1=P  carry=CIN  dbl_ovrd=off  block_type=constant
      settings: block_version=10.1.2
  Constant1 : xbsIndex_r4/Constant  arith_type=Boolean  const=0  n_bits=4  bin_pt=0  explicit_period=off  period=1  equ=P=C  opselect=C  inp2=PCIN>>17  opr=+  inp1=P  carry=CIN  dbl_ovrd=off  block_type=constant
      settings: block_version=10.1.2
  ROM : xbsIndex_r4/ROM  depth=numSubcarriers*3 %AntA, AntB, BaseRate  initVector=subcarrier_QAM_Values  distributed_mem=Block RAM  rst=off  init_reg=0  en=off  latency=1  arith_type=Unsigned  n_bits=4  bin_pt=0  dbl_ovrd=off  optimize=Area  use_rpm=on  block_type=sprom
      settings: block_version=10.1
  Shared Memory : xbsIndex_r4/Shared Memory  shared_memory_name='RxModulation'  depth=numSubcarriers*3 %AntA, AntB, BaseRate  ownership=Locally Owned and Initialized  initVector=subcarrier_QAM_Values  mutex=Unprotected  mode=Read and Write  write_mode=Read After Write  time_out=0  latency=1  explicit_data_type=on  arith_type=Unsigned  n_bits=4  bin_pt=0  implementation=Block RAM  use_rpm=off  block_type=shmem
      settings: AttributesFormatString=<< %<shared_memory_name> >>  block_version=10.1.2
  ModMask : Outport
  wires:
  Constant/1 -> Shared Memory/2
  Constant1/1 -> Shared Memory/3
  Addr/1 -> Shared Memory/1, ROM/1
  Shared Memory/1 -> ModMask/1

== system ofdm_txrx_mimo_2x/OFDM Rx MIMO/Equalizer & Packetizer/Packet_Constructor/Pkt Interrupt Outputs/Pkt Interrupt Control ==
  Rx _Int_goodPkt{1..6} (6 alike) : xbsIndex_r4/Gateway Out  hdl_port=off  timing_constraint=None  locs_specified=off  LOCs={}  block_type=gatewayout
      settings: infoedit="Gateway out block.  Converts Xilinx fixed point inputs into ouputs of type Simulink integer, double, or fixed point.<P><P>Hardware notes:  In hardware these blocks become top level output ports or are discarded, depending on how they are configured."  xl_area=[0 0 0 0 1 0 0]  block_version=VER_STRING_GOES_HERE
  RxIntrControl : Scope  NumInputPorts=6
      settings: YMin=-1~-5~-5~-5~-5~-5  YMax=1~5~5~5~5~5  SaveName=ScopeData59
  GoodPkt_En : Outport
  PacketInts_Rst : Outport  Port=2
  BadPkt_En : Outport  Port=3
  HeaderInts_Rst : Outport  Port=4
  GoodHeader_En : Outport  Port=5
  BadHeader_En : Outport  Port=6
  wires:
  [intrCtrl_RxPktInterrupts_rst] -> PacketInts_Rst/1, Rx _Int_goodPkt2/1
  [intrCtrl_RxHeaderInterrupts_rst] -> HeaderInts_Rst/1, Rx _Int_goodPkt3/1
  [intrCtrl_RxGoodPkt_en] -> GoodPkt_En/1, Rx _Int_goodPkt4/1
  [intrCtrl_RxBadPkt_en] -> BadPkt_En/1, Rx _Int_goodPkt5/1
  [intrCtrl_RxGoodHeader_en] -> GoodHeader_En/1, Rx _Int_goodPkt1/1
  [intrCtrl_RxBadHeader_en] -> BadHeader_En/1, Rx _Int_goodPkt6/1
  Rx _Int_goodPkt2/1 -> RxIntrControl/1
  Rx _Int_goodPkt3/1 -> RxIntrControl/2
  Rx _Int_goodPkt4/1 -> RxIntrControl/3
  Rx _Int_goodPkt5/1 -> RxIntrControl/4
  Rx _Int_goodPkt1/1 -> RxIntrControl/5
  Rx _Int_goodPkt6/1 -> RxIntrControl/6

== system ofdm_txrx_mimo_2x/OFDM Rx MIMO/Equalizer & Packetizer/Phase Noise Tracker/Coarse Tracking/Times_Alpha ==
  note: We found in hardware that a value of 0.5
      | always worked, so we optimized away
      | the constant and multiplier altogether
  M : Inport
  Mult_0.5 : xbsIndex_r4/Scale  scale_factor=-1  dbl_ovrd=off  block_type=scale
      settings: infoedit=Scales input by a power of two by adjusting the binary point position.<P><P>Hardware notes: In hardware this block costs nothing.  block_version=8.2.01
  P : Outport
  wires:
  Mult_0.5/1 -> P/1
  M/1 -> Mult_0.5/1

== system ofdm_txrx_mimo_2x/OFDM Rx MIMO/Equalizer & Packetizer/Phase Noise Tracker/Fine Tracking/Times_Beta ==
  note: We found in hardware that a value of 0.5
      | always worked, so we optimized away
      | the constant and multiplier altogether
  M : Inport
  Mult_0.5 : xbsIndex_r4/Scale  scale_factor=-1  dbl_ovrd=off  block_type=scale
      settings: infoedit=Scales input by a power of two by adjusting the binary point position.<P><P>Hardware notes: In hardware this block costs nothing.  block_version=8.2.01
  P : Outport
  wires:
  Mult_0.5/1 -> P/1
  M/1 -> Mult_0.5/1

== system ofdm_txrx_mimo_2x/OFDM Rx MIMO/Equalizer & Packetizer/Phase Noise Tracker/Fine Tracking/Times_Gamma ==
  note: We found in hardware that a value of 0.5
      | always worked, so we optimized away
      | the constant and multiplier altogether
  M : Inport
  Mult_0.5 : xbsIndex_r4/Scale  scale_factor=-1  dbl_ovrd=off  block_type=scale
      settings: infoedit=Scales input by a power of two by adjusting the binary point position.<P><P>Hardware notes: In hardware this block costs nothing.  block_version=8.2.01
  P : Outport
  wires:
  M/1 -> Mult_0.5/1
  Mult_0.5/1 -> P/1
